simulink model "V2x_TC_IntelligentTrafficSigns_cs"  (Simulink 7.6)
settings: Solver=FixedStepDiscrete  SolverMode=SingleTasking  StartTime=0.0  StopTime=66  FixedStep=1/100  RelTol=1e-3
PreLoadFcn:
  | check_NUTS_defined
  | 
  | assignin('base',['model_closed_' bdroot],false);addpath('../includes_V2x');
InitFcn:
  | PreScanExperimentInitFcn(bdroot)
StopFcn:
  | PreScanExperimentStopFcn(bdroot);
  | 
undefined symbols (not defined in the file or in its folder): acceleration, actorID, actorType, DiscIntNeverNeededParam, GPS_alt, GPS_lat, GPS_long, lightBarInUse, messageID, Newton, publicActorType, Raphson, rotZ, sigbuilder_block, sireneInUse, trafficLightPriority, turnAdvice, velocity, x_ini_VRML, y_ini_VRML, yawRate, z_ini_VRML
libraries: simulink x111, PreScanSimulinkLibrary x7, simulink_extras x4, PreScanUsefulBlocks x3
(part 15 of 16)

== system V2x_TC_IntelligentTrafficSigns_cs/TS_Tcross_2/Infrastructure CAM generator/CAM generation/no ==
  Action Port : ActionPort  PropagateVarSize=During execution  ActionType=else
  Constant : Constant  Value=0
  Out1 : Outport  OutputWhenDisabled=reset  InitialOutput=0
  wires:
  Constant/1 -> Out1/1

== system V2x_TC_IntelligentTrafficSigns_cs/TS_Tcross_2/Infrastructure CAM generator/CAM generation/yes ==
  Action Port : ActionPort  PropagateVarSize=During execution  ActionType=then
  Constant : Constant
  Data Store Write5 : DataStoreWrite  DataStoreName=CAM_time
  Digital Clock1 : DigitalClock  SampleTime=0.01
  Out1 : Outport  OutputWhenDisabled=reset  InitialOutput=0
  wires:
  Digital Clock1/1 -> Data Store Write5/1
  Constant/1 -> Out1/1

== system V2x_TC_IntelligentTrafficSigns_cs/TS_Tcross_3/Infrastructure CAM generator/CAM generation/no ==
  Action Port : ActionPort  PropagateVarSize=During execution  ActionType=else
  Constant : Constant  Value=0
  Out1 : Outport  OutputWhenDisabled=reset  InitialOutput=0
  wires:
  Constant/1 -> Out1/1

== system V2x_TC_IntelligentTrafficSigns_cs/TS_Tcross_3/Infrastructure CAM generator/CAM generation/yes ==
  Action Port : ActionPort  PropagateVarSize=During execution  ActionType=then
  Constant : Constant
  Data Store Write5 : DataStoreWrite  DataStoreName=CAM_time
  Digital Clock1 : DigitalClock  SampleTime=0.01
  Out1 : Outport  OutputWhenDisabled=reset  InitialOutput=0
  wires:
  Digital Clock1/1 -> Data Store Write5/1
  Constant/1 -> Out1/1

== system V2x_TC_IntelligentTrafficSigns_cs/TS_end_restrictions/Infrastructure CAM generator/CAM generation/no ==
  Action Port : ActionPort  PropagateVarSize=During execution  ActionType=else
  Constant : Constant  Value=0
  Out1 : Outport  OutputWhenDisabled=reset  InitialOutput=0
  wires:
  Constant/1 -> Out1/1

== system V2x_TC_IntelligentTrafficSigns_cs/TS_end_restrictions/Infrastructure CAM generator/CAM generation/yes ==
  Action Port : ActionPort  PropagateVarSize=During execution  ActionType=then
  Constant : Constant
  Data Store Write5 : DataStoreWrite  DataStoreName=CAM_time
  Digital Clock1 : DigitalClock  SampleTime=0.01
  Out1 : Outport  OutputWhenDisabled=reset  InitialOutput=0
  wires:
  Digital Clock1/1 -> Data Store Write5/1
  Constant/1 -> Out1/1

== system V2x_TC_IntelligentTrafficSigns_cs/TS_end_restrictions_r/Infrastructure CAM generator/CAM generation/no ==
  Action Port : ActionPort  PropagateVarSize=During execution  ActionType=else
  Constant : Constant  Value=0
  Out1 : Outport  OutputWhenDisabled=reset  InitialOutput=0
  wires:
  Constant/1 -> Out1/1

== system V2x_TC_IntelligentTrafficSigns_cs/TS_end_restrictions_r/Infrastructure CAM generator/CAM generation/yes ==
  Action Port : ActionPort  PropagateVarSize=During execution  ActionType=then
  Constant : Constant
  Data Store Write5 : DataStoreWrite  DataStoreName=CAM_time
  Digital Clock1 : DigitalClock  SampleTime=0.01
  Out1 : Outport  OutputWhenDisabled=reset  InitialOutput=0
  wires:
  Digital Clock1/1 -> Data Store Write5/1
  Constant/1 -> Out1/1

== system V2x_TC_IntelligentTrafficSigns_cs/TS_no_entry/Infrastructure CAM generator/CAM generation/no ==
  Action Port : ActionPort  PropagateVarSize=During execution  ActionType=else
  Constant : Constant  Value=0
  Out1 : Outport  OutputWhenDisabled=reset  InitialOutput=0
  wires:
  Constant/1 -> Out1/1

== system V2x_TC_IntelligentTrafficSigns_cs/TS_no_entry/Infrastructure CAM generator/CAM generation/yes ==
  Action Port : ActionPort  PropagateVarSize=During execution  ActionType=then
  Constant : Constant
  Data Store Write5 : DataStoreWrite  DataStoreName=CAM_time
  Digital Clock1 : DigitalClock  SampleTime=0.01
  Out1 : Outport  OutputWhenDisabled=reset  InitialOutput=0
  wires:
  Digital Clock1/1 -> Data Store Write5/1
  Constant/1 -> Out1/1

== system V2x_TC_IntelligentTrafficSigns_cs/TS_v50_1/Infrastructure CAM generator/CAM generation/no ==
  Action Port : ActionPort  PropagateVarSize=During execution  ActionType=else
  Constant : Constant  Value=0
  Out1 : Outport  OutputWhenDisabled=reset  InitialOutput=0
  wires:
  Constant/1 -> Out1/1

== system V2x_TC_IntelligentTrafficSigns_cs/TS_v50_1/Infrastructure CAM generator/CAM generation/yes ==
  Action Port : ActionPort  PropagateVarSize=During execution  ActionType=then
  Constant : Constant
  Data Store Write5 : DataStoreWrite  DataStoreName=CAM_time
  Digital Clock1 : DigitalClock  SampleTime=0.01
  Out1 : Outport  OutputWhenDisabled=reset  InitialOutput=0
  wires:
  Digital Clock1/1 -> Data Store Write5/1
  Constant/1 -> Out1/1

== system V2x_TC_IntelligentTrafficSigns_cs/TS_v50_2/Infrastructure CAM generator/CAM generation/no ==
  Action Port : ActionPort  PropagateVarSize=During execution  ActionType=else
  Constant : Constant  Value=0
  Out1 : Outport  OutputWhenDisabled=reset  InitialOutput=0
  wires:
  Constant/1 -> Out1/1

== system V2x_TC_IntelligentTrafficSigns_cs/TS_v50_2/Infrastructure CAM generator/CAM generation/yes ==
  Action Port : ActionPort  PropagateVarSize=During execution  ActionType=then
  Constant : Constant
  Data Store Write5 : DataStoreWrite  DataStoreName=CAM_time
  Digital Clock1 : DigitalClock  SampleTime=0.01
  Out1 : Outport  OutputWhenDisabled=reset  InitialOutput=0
  wires:
  Digital Clock1/1 -> Data Store Write5/1
  Constant/1 -> Out1/1

== system V2x_TC_IntelligentTrafficSigns_cs/TS_v50_3/Infrastructure CAM generator/CAM generation/no ==
  Action Port : ActionPort  PropagateVarSize=During execution  ActionType=else
  Constant : Constant  Value=0
  Out1 : Outport  OutputWhenDisabled=reset  InitialOutput=0
  wires:
  Constant/1 -> Out1/1

== system V2x_TC_IntelligentTrafficSigns_cs/TS_v50_3/Infrastructure CAM generator/CAM generation/yes ==
  Action Port : ActionPort  PropagateVarSize=During execution  ActionType=then
  Constant : Constant
  Data Store Write5 : DataStoreWrite  DataStoreName=CAM_time
  Digital Clock1 : DigitalClock  SampleTime=0.01
  Out1 : Outport  OutputWhenDisabled=reset  InitialOutput=0
  wires:
  Digital Clock1/1 -> Data Store Write5/1
  Constant/1 -> Out1/1

== system V2x_TC_IntelligentTrafficSigns_cs/TS_v80_1/Infrastructure CAM generator/CAM generation/no ==
  Action Port : ActionPort  PropagateVarSize=During execution  ActionType=else
  Constant : Constant  Value=0
  Out1 : Outport  OutputWhenDisabled=reset  InitialOutput=0
  wires:
  Constant/1 -> Out1/1

== system V2x_TC_IntelligentTrafficSigns_cs/TS_v80_1/Infrastructure CAM generator/CAM generation/yes ==
  Action Port : ActionPort  PropagateVarSize=During execution  ActionType=then
  Constant : Constant
  Data Store Write5 : DataStoreWrite  DataStoreName=CAM_time
  Digital Clock1 : DigitalClock  SampleTime=0.01
  Out1 : Outport  OutputWhenDisabled=reset  InitialOutput=0
  wires:
  Digital Clock1/1 -> Data Store Write5/1
  Constant/1 -> Out1/1

== system V2x_TC_IntelligentTrafficSigns_cs/TS_v80_3/Infrastructure CAM generator/CAM generation/no ==
  Action Port : ActionPort  PropagateVarSize=During execution  ActionType=else
  Constant : Constant  Value=0
  Out1 : Outport  OutputWhenDisabled=reset  InitialOutput=0
  wires:
  Constant/1 -> Out1/1

== system V2x_TC_IntelligentTrafficSigns_cs/TS_v80_3/Infrastructure CAM generator/CAM generation/yes ==
  Action Port : ActionPort  PropagateVarSize=During execution  ActionType=then
  Constant : Constant
  Data Store Write5 : DataStoreWrite  DataStoreName=CAM_time
  Digital Clock1 : DigitalClock  SampleTime=0.01
  Out1 : Outport  OutputWhenDisabled=reset  InitialOutput=0
  wires:
  Digital Clock1/1 -> Data Store Write5/1
  Constant/1 -> Out1/1

== system V2x_TC_IntelligentTrafficSigns_cs/TS_v80_4/Infrastructure CAM generator/CAM generation/no ==
  Action Port : ActionPort  PropagateVarSize=During execution  ActionType=else
  Constant : Constant  Value=0
  Out1 : Outport  OutputWhenDisabled=reset  InitialOutput=0
  wires:
  Constant/1 -> Out1/1

== system V2x_TC_IntelligentTrafficSigns_cs/TS_v80_4/Infrastructure CAM generator/CAM generation/yes ==
  Action Port : ActionPort  PropagateVarSize=During execution  ActionType=then
  Constant : Constant
  Data Store Write5 : DataStoreWrite  DataStoreName=CAM_time
  Digital Clock1 : DigitalClock  SampleTime=0.01
  Out1 : Outport  OutputWhenDisabled=reset  InitialOutput=0
  wires:
  Digital Clock1/1 -> Data Store Write5/1
  Constant/1 -> Out1/1

== system V2x_TC_IntelligentTrafficSigns_cs/BMW_X5_1/Traffic Controller/Intersection warning controller/Enabled Subsystem/Intersection ==
  hostRotZ : Inport
  hostTurnAdvice : Inport  Port=2
  host GPS lat [deg] : Inport  Port=3
  host GPS long [deg] : Inport  Port=4
  host GPS alt [m] : Inport  Port=5
  GPS_lat : Inport  Port=6
  GPS_long : Inport  Port=7
  GPS_alt : Inport  Port=8
  targetVelocity : Inport  Port=9
  turnAdvice : Inport  Port=10
  rotZ : Inport  Port=11
  actorType : Inport  Port=12
  crossingType : Inport  Port=13
  crossing detection range : Inport  Port=14
  Closest crossing selection : Subsystem
  GPS to XYZ difference (vectorized) : Subsystem
  GPS_alt_1 : InportShadow  Port=8
  GPS_lat_1 : InportShadow  Port=6
  GPS_long_1 : InportShadow  Port=7
  Intersection collision warning controller : Subsystem
  Terminator_2 : Terminator
  cartesian 2 cylindrical : Subsystem
  crossing detection range_1 : InportShadow  Port=14
  hostRotZ_1 : InportShadow  Port=1
  relative heading : Subsystem
  warning left lane : Outport
  warning straight lane : Outport  Port=2
  warning right lane : Outport  Port=3
  wires:
  host GPS lat [deg]/1 -> Closest crossing selection/2(host GPS lat)
  host GPS long [deg]/1 -> Closest crossing selection/3(host GPS long)
  host GPS alt [m]/1 -> Closest crossing selection/4(host GPS alt)
  Closest crossing selection/1(crossing GPS lat) -> GPS to XYZ difference (vectorized)/1(hostLat)
  Closest crossing selection/2(crossing GPS long) -> GPS to XYZ difference (vectorized)/2(hostLong)
  Closest crossing selection/3(crossing GPS alt) -> GPS to XYZ difference (vectorized)/3(hostAlt)
  crossing detection range/1 -> Closest crossing selection/8(crossing detection range)
  crossing detection range_1/1 -> Intersection collision warning controller/3(crossing detection range)
  hostRotZ/1 -> relative heading/2(hostRotZ)
  hostTurnAdvice/1 -> Intersection collision warning controller/2(hostTurnAdvice)
  cartesian 2 cylindrical/1(range) -> Intersection collision warning controller/6(target range)
  relative heading/1(dRotZ) -> Intersection collision warning controller/8(target heading)
  Intersection collision warning controller/1(warning left lane) -> warning left lane/1
  Intersection collision warning controller/2(warning straight lane) -> warning straight lane/1
  Intersection collision warning controller/3(warning right lane) -> warning right lane/1
  GPS to XYZ difference (vectorized)/1(dX) -> cartesian 2 cylindrical/1(dX)
  GPS to XYZ difference (vectorized)/2(dY) -> cartesian 2 cylindrical/2(dY)
  GPS_lat/1 -> Closest crossing selection/5(GPS lat)
  GPS_long/1 -> Closest crossing selection/6(GPS long)
  GPS_alt/1 -> Closest crossing selection/7(GPS alt)
  crossingType/1 -> Closest crossing selection/1(crossingType)
  rotZ/1 -> relative heading/1(rotZ)
  GPS_lat_1/1 -> GPS to XYZ difference (vectorized)/4(lat)
  GPS_long_1/1 -> GPS to XYZ difference (vectorized)/5(long)
  GPS_alt_1/1 -> GPS to XYZ difference (vectorized)/6(alt)
  turnAdvice/1 -> Intersection collision warning controller/4(target intention)
  targetVelocity/1 -> Intersection collision warning controller/5(target velocity)
  actorType/1 -> Intersection collision warning controller/1(actorType)
  GPS to XYZ difference (vectorized)/3(dZ) -> Terminator_2/1
  hostRotZ_1/1 -> cartesian 2 cylindrical/3(hostRotZ)
  cartesian 2 cylindrical/2(angle) -> Intersection collision warning controller/7(target angle)

== system V2x_TC_IntelligentTrafficSigns_cs/BMW_X5_1/Traffic Controller/Intersection warning controller/Enabled Subsystem/sort using ID ==
  IDs : Inport
  in1 : Inport  Port=2
  in2 : Inport  Port=3
  in3 : Inport  Port=4
  in4 : Inport  Port=5
  in5 : Inport  Port=6
  in6 : Inport  Port=7
  in7 : Inport  Port=8
  in8 : Inport  Port=9
  Embedded MATLAB Function : Stateflow chart  TreatAsAtomicUnit=on
  Selector{1..8} (8 alike) : Selector  IndexOptions=Index vector (port)  Indices=[1 3]  OutputSizes=1
  Out1 : Outport
  Out2 : Outport  Port=2
  Out3 : Outport  Port=3
  Out4 : Outport  Port=4
  Out5 : Outport  Port=5
  Out6 : Outport  Port=6
  Out7 : Outport  Port=7
  Out8 : Outport  Port=8
  wires:
  Embedded MATLAB Function/1(y) -> Selector7/2, Selector8/2, Selector6/2, Selector5/2, Selector4/2, Selector3/2, Selector2/2, Selector1/2
  IDs/1 -> Embedded MATLAB Function/1(ids)
  in7/1 -> Selector7/1
  in6/1 -> Selector6/1
  Selector7/1 -> Out7/1
  Selector6/1 -> Out6/1
  in5/1 -> Selector5/1
  in4/1 -> Selector4/1
  in3/1 -> Selector3/1
  in2/1 -> Selector2/1
  Selector5/1 -> Out5/1
  Selector4/1 -> Out4/1
  Selector3/1 -> Out3/1
  Selector2/1 -> Out2/1
  Selector1/1 -> Out1/1
  in1/1 -> Selector1/1
  in8/1 -> Selector8/1
  Selector8/1 -> Out8/1

== system V2x_TC_IntelligentTrafficSigns_cs/BMW_X5_1/Traffic Controller/Road accident detection controller/Compute distance to road accident/If Action Subsystem ==
  conditions : Inport
  GPS lat1 : Inport  Port=2
  GPS long1 : Inport  Port=3
  GPS alt1 : Inport  Port=4
  host_GPS_lat : Inport  Port=5
  host_GPS_long : Inport  Port=6
  host_GPS_alt : Inport  Port=7
  Action Port : ActionPort  PropagateVarSize=During execution  ActionType=then
  GPS to distance : Subsystem
  Product7 : Product  RndMeth=Zero
  Product8 : Product  RndMeth=Zero
  Product9 : Product  RndMeth=Zero
  Sum6 : Sum  Inputs=+
  Sum7 : Sum  Inputs=+
  Sum8 : Sum  Inputs=+
  distance : Outport  InitialOutput=0
  wires:
  conditions/1 -> Product7/1, Product9/1, Product8/1
  GPS lat1/1 -> Product7/2
  GPS long1/1 -> Product8/2
  GPS alt1/1 -> Product9/2
  Product7/1 -> Sum6/1
  Product8/1 -> Sum7/1
  Product9/1 -> Sum8/1
  Sum6/1 -> GPS to distance/4(lat)
  Sum7/1 -> GPS to distance/5(long)
  Sum8/1 -> GPS to distance/6(alt)
  host_GPS_lat/1 -> GPS to distance/1(hostLat)
  host_GPS_long/1 -> GPS to distance/2(hostLong)
  host_GPS_alt/1 -> GPS to distance/3(hostAlt)
  GPS to distance/1(dist) -> distance/1

== system V2x_TC_IntelligentTrafficSigns_cs/BMW_X5_1/Traffic Controller/Road accident detection controller/Compute distance to road accident/If Action Subsystem1 ==
  Action Port : ActionPort  PropagateVarSize=During execution  ActionType=else
  Constant : Constant  Value=0
  distance : Outport  InitialOutput=0
  wires:
  Constant/1 -> distance/1

== system V2x_TC_IntelligentTrafficSigns_cs/BMW_X5_1/Traffic Controller/Traffic signs and  traffic lights controller/Enabled Subsystem/GPS to XYZ difference (vectorized) ==
  hostLat : Inport
  hostLong : Inport  Port=2
  hostAlt : Inport  Port=3
  lat : Inport  Port=4
  long : Inport  Port=5
  alt : Inport  Port=6
  Add : Sum  Inputs=+-
  Add1 : Sum  Inputs=+-
  Add2 : Sum  Inputs=+-
  Add3 : Sum  Inputs=+++
  Product{,1..4} (5 alike) : Product  RndMeth=Zero
  d2r : Gain  Gain=pi/180
  d2r1 : Gain  Gain=pi/180
  mean Earth radius (latitudal) : Constant  Value=6335439.8775
  mean Earth radius (longitudal) : Constant  Value=6378137.55
  dist_sq : Outport
  dX : Outport  Port=2
  dY : Outport  Port=3
  dZ : Outport  Port=4
  wires:
  Add3/1 -> dist_sq/1
  Product4/1 -> Add3/3
  Product3/1 -> Add3/2
  Product/1 -> Add3/1
  Add2/1 -> Product4/1, Product4/2, dZ/1
  Product1/1 -> Product3/2, Product3/1, dX/1
  Product2/1 -> Product/2, Product/1, dY/1
  mean Earth radius (latitudal)/1 -> Product2/2
  mean Earth radius (longitudal)/1 -> Product1/2
  d2r1/1 -> Product1/1
  d2r/1 -> Product2/1
  Add1/1 -> d2r1/1
  Add/1 -> d2r/1
  hostAlt/1 -> Add2/2
  alt/1 -> Add2/1
  hostLong/1 -> Add1/2
  long/1 -> Add1/1
  hostLat/1 -> Add/2
  lat/1 -> Add/1

== system V2x_TC_IntelligentTrafficSigns_cs/BMW_X5_1/Traffic Controller/Traffic signs and  traffic lights controller/Enabled Subsystem/distance condition ==
  distance_squared : Inport
  hostVelocity : Inport  Port=2
  If : If  IfExpression=u1 ~= 0
  If Action Subsystem : Subsystem  TreatAsAtomicUnit=on
  If Action Subsystem1 : Subsystem  TreatAsAtomicUnit=on
  Merge : Merge
  Product3 : Product  RndMeth=Zero
  Product4 : Product  RndMeth=Zero
  Relational Operator1 : RelationalOperator  Operator=<  OutDataTypeStr=boolean
  headway time : Constant  Value=3
  dist_cond : Outport
  wires:
  Product3/1 -> If Action Subsystem1/1(headway time), Relational Operator1/2
  distance_squared/1 -> If Action Subsystem1/2(d2), If Action Subsystem/1(d2)
  Relational Operator1/1 -> dist_cond/1
  Merge/1 -> Relational Operator1/1
  If Action Subsystem1/1(t2) -> Merge/2
  If Action Subsystem/1(t2) -> Merge/1
  Product4/1 -> If Action Subsystem/2(v2)
  If/2 -> If Action Subsystem1/Action
  If/1 -> If Action Subsystem/Action
  hostVelocity/1 -> Product4/2, Product4/1, If/1
  headway time/1 -> Product3/1, Product3/2

== system V2x_TC_IntelligentTrafficSigns_cs/BMW_X5_1/Traffic Controller/Traffic signs and  traffic lights controller/Enabled Subsystem/heading condition ==
  hostRotZ : Inport
  rotZ : Inport  Port=2
  Abs : Abs
  Add : Sum  Inputs=+-
  Add1 : Sum  Inputs=-+
  Bias : Bias  Bias=180
  Constant : Constant  Value=360
  Constant1 : Constant  Value=360
  Logical Operator : Logic  Operator=OR  AllPortsSameDT=off  OutDataTypeStr=boolean
  Math Function : Math  Operator=mod  SaturateOnIntegerOverflow=on
  Relational Operator : RelationalOperator  Operator=<  OutDataTypeStr=boolean
  Relational Operator1 : RelationalOperator  Operator=>  OutDataTypeStr=boolean
  heading difference : Constant  Value=45
  head_cond : Outport
  wires:
  Logical Operator/1 -> head_cond/1
  Relational Operator1/1 -> Logical Operator/2
  Relational Operator/1 -> Logical Operator/1
  Constant1/1 -> Add1/2
  Add1/1 -> Relational Operator1/2
  heading difference/1 -> Add1/1, Relational Operator/2
  Abs/1 -> Relational Operator1/1, Relational Operator/1
  Math Function/1 -> Abs/1
  hostRotZ/1 -> Add/2
  rotZ/1 -> Add/1
  Bias/1 -> Math Function/1
  Constant/1 -> Math Function/2
  Add/1 -> Bias/1

== system V2x_TC_IntelligentTrafficSigns_cs/BMW_X5_1/Traffic Controller/Traffic signs and  traffic lights controller/Enabled Subsystem/major object selection lights ==
  conditions : Inport
  messagesID : Inport  Port=2
  traffic lights priorities : Inport  Port=3
  If : If  IfExpression=u1
  If Action Subsystem : Subsystem  TreatAsAtomicUnit=on
  If Action Subsystem1 : Subsystem  TreatAsAtomicUnit=on
  Logical Operator1 : Logic  Operator=OR  Inputs=1  AllPortsSameDT=off  OutDataTypeStr=boolean
  Merge : Merge
  Merge2 : Merge
  Traffic Lights Warning : Subsystem
  Warning : Outport
  MessageID : Outport  Port=2
  Priority : Outport  Port=3
  wires:
  Traffic Lights Warning/1(Warning) -> Warning/1
  Merge/1 -> Traffic Lights Warning/1(trafficLightPriority), Priority/1
  Merge2/1 -> MessageID/1
  If Action Subsystem/2(Priority) -> Merge/1
  If Action Subsystem/1(Message) -> Merge2/1
  If Action Subsystem1/1(Message) -> Merge2/2
  If Action Subsystem1/2(Priority) -> Merge/2
  traffic lights priorities/1 -> If Action Subsystem/3(traffic lights priorities)
  messagesID/1 -> If Action Subsystem/2(messagesID)
  conditions/1 -> If Action Subsystem/1(cond), Logical Operator1/1
  If/2 -> If Action Subsystem1/Action
  If/1 -> If Action Subsystem/Action
  Logical Operator1/1 -> If/1

== system V2x_TC_IntelligentTrafficSigns_cs/BMW_X5_1/Traffic Controller/Traffic signs and  traffic lights controller/Enabled Subsystem/major object selection signs ==
  conditions : Inport
  hostVelocity : Inport  Port=2
  messagesID : Inport  Port=3
  If : If  IfExpression=u1
  If Action Subsystem : Subsystem  TreatAsAtomicUnit=on
  If Action Subsystem1 : Subsystem  TreatAsAtomicUnit=on
  Logical Operator1 : Logic  Operator=OR  Inputs=1  AllPortsSameDT=off  OutDataTypeStr=boolean
  Merge : Merge
  Merge1 : Merge
  Warning : Outport
  MessageID : Outport  Port=2
  wires:
  hostVelocity/1 -> If Action Subsystem/3(hostVelocity)
  Merge/1 -> MessageID/1
  Merge1/1 -> Warning/1
  If Action Subsystem1/1(Warning) -> Merge1/2
  If Action Subsystem/1(Warning) -> Merge1/1
  If Action Subsystem/2(Message) -> Merge/1
  If Action Subsystem1/2(Message) -> Merge/2
  messagesID/1 -> If Action Subsystem/2(messagesID)
  conditions/1 -> If Action Subsystem/1(cond), Logical Operator1/1
  If/2 -> If Action Subsystem1/Action
  If/1 -> If Action Subsystem/Action
  Logical Operator1/1 -> If/1

== system V2x_TC_IntelligentTrafficSigns_cs/BMW_X5_1/Traffic Controller/Traffic signs and  traffic lights controller/Enabled Subsystem/orientation condition ==
  hostRotZ : Inport
  dX : Inport  Port=2
  dY : Inport  Port=3
  dZ : Inport  Port=4
  Abs : Abs
  Gain : Gain  Gain=pi/180
  Relational Operator : RelationalOperator  Operator=<  OutDataTypeStr=boolean
  Terminator : Terminator
  Trigonometric Function : Trigonometry  Operator=atan2
  d2r : Gain  Gain=pi/180
  orientation limit : Constant  Value=45
  to local reference system : Subsystem
  orient_cond : Outport
  wires:
  to local reference system/2(dx) -> Trigonometric Function/2
  to local reference system/1(dy) -> Trigonometric Function/1
  dY/1 -> to local reference system/3(dY)
  dX/1 -> to local reference system/2(dX)
  Gain/1 -> to local reference system/1(rotZ)
  hostRotZ/1 -> Gain/1
  Trigonometric Function/1 -> Abs/1
  Relational Operator/1 -> orient_cond/1
  d2r/1 -> Relational Operator/2
  Abs/1 -> Relational Operator/1
  orientation limit/1 -> d2r/1
  dZ/1 -> Terminator/1

== system V2x_TC_IntelligentTrafficSigns_cs/BMW_X5_1/Traffic Controller/Traffic signs and  traffic lights controller/Enabled Subsystem/right road side condition ==
  hostRotZ : Inport
  dX : Inport  Port=2
  dY : Inport  Port=3
  Compare To Zero : simulink/Logic and Bit Operations/Compare To Zero  SystemSampleTime=-1  relop=<  LogicOutDataTypeMode=boolean
  Gain : Gain  Gain=pi/180
  to local reference system : Subsystem
  orient_cond : Outport
  wires:
  Compare To Zero/1 -> orient_cond/1
  hostRotZ/1 -> Gain/1
  Gain/1 -> to local reference system/1(rotZ)
  dX/1 -> to local reference system/2(dX)
  dY/1 -> to local reference system/3(dY)
  to local reference system/1(dy) -> Compare To Zero/1

== system V2x_TC_IntelligentTrafficSigns_cs/BMW_X5_1/Trajectories/ElapsedTrack1/MuxState/Substitute 90 & 270 deg (and its plurals) ==
  Angle [rad] : Inport
  270 deg detection : simulink/Model Verification/Check  Static Gap  Description=u <= 269.5 || 270.5 <= u  SystemSampleTime=-1  max=270.5  max_included=on  min=269.5  min_included=on  enabled=on  stopWhenAssertionFail=off  export=on  icon=graphic
  90 deg detection : simulink/Model Verification/Check  Static Gap  Description=u <= 89.5 || 90.5 <= u  SystemSampleTime=-1  max=90.5  max_included=on  min=89.5  min_included=on  enabled=on  stopWhenAssertionFail=off  export=on  icon=graphic
  Angle 0-360 deg limiter : Subsystem
  Degrees to Radians : simulink_extras/Transformations/Degrees to Radians  SystemSampleTime=-1
  Radians to Degrees : simulink_extras/Transformations/Radians to Degrees  SystemSampleTime=-1
  Substitution  Value : Constant  Value=269.5
  Substitution Value : Constant  Value=89.5
  Switch : Switch  Threshold=0.5
  Switch1 : Switch  Threshold=0.5
  Subst Angle [rad] : Outport
  wires:
  Angle [rad]/1 -> Radians to Degrees/1
  Degrees to Radians/1 -> Subst Angle [rad]/1
  Radians to Degrees/1 -> Angle 0-360 deg limiter/1(Angle [deg])
  Switch1/1 -> Degrees to Radians/1
  Substitution  Value/1 -> Switch1/3
  270 deg detection/1 -> Switch1/2
  Switch/1 -> Switch1/1, 270 deg detection/1
  Substitution Value/1 -> Switch/3
  90 deg detection/1 -> Switch/2
  Angle 0-360 deg limiter/1(LimAngle [deg]) -> 90 deg detection/1, Switch/1

== system V2x_TC_IntelligentTrafficSigns_cs/BMW_X5_1/Trajectories/Traj2State1/Traj2State2/MuxState ==
  x [m] : Inport
  y [m] : Inport  Port=2
  z [m] : Inport  Port=3
  dx [m/s] : Inport  Port=4
  dy [m/s] : Inport  Port=5
  dz [m/s] : Inport  Port=6
  R [rad] : Inport  Port=7
  P [rad] : Inport  Port=8
  Y [rad] : Inport  Port=9
  Mux : Mux  Inputs=9
  state : Outport
  wires:
  z [m]/1 -> Mux/3
  y [m]/1 -> Mux/2
  x [m]/1 -> Mux/1
  Y [rad]/1 -> Mux/9
  P [rad]/1 -> Mux/8
  R [rad]/1 -> Mux/7
  dz [m/s]/1 -> Mux/6
  dy [m/s]/1 -> Mux/5
  dx [m/s]/1 -> Mux/4
  Mux/1 -> state/1

== system V2x_TC_IntelligentTrafficSigns_cs/BMW_X5_1/Trajectories/Traj2State1/Traj2State2/Traj2MuxState_Ext ==
  Traj : Inport
  Terminator{1..9} (9 alike) : Terminator
  demux : Subsystem
  x [m] : Outport
  y [m] : Outport  Port=2
  z [m] : Outport  Port=3
  dx [m/s] : Outport  Port=4
  dy [m/s] : Outport  Port=5
  dz [m/s] : Outport  Port=6
  R [rad] : Outport  Port=7
  P [rad] : Outport  Port=8
  Y [rad] : Outport  Port=9
  wires:
  demux/9(Y) -> Y [rad]/1
  demux/7(R) -> R [rad]/1
  demux/5(vely) -> dy [m/s]/1
  demux/4(velx) -> dx [m/s]/1
  demux/10(velR) -> Terminator4/1
  demux/11(velP) -> Terminator5/1
  demux/12(velY) -> Terminator1/1
  demux/13(accx) -> Terminator8/1
  demux/14(accy) -> Terminator9/1
  demux/15(accz) -> Terminator7/1
  demux/18(accY) -> Terminator2/1
  demux/16(accR) -> Terminator3/1
  demux/17(accP) -> Terminator6/1
  demux/3(z) -> z [m]/1
  Traj/1 -> demux/1(traj)
  demux/6(velz) -> dz [m/s]/1
  demux/1(x) -> x [m]/1
  demux/2(y) -> y [m]/1
  demux/8(P) -> P [rad]/1

== system V2x_TC_IntelligentTrafficSigns_cs/BMW_X5_1/vehicle CAM generator/CAM generation/maybe/If Action Subsystem ==
  x [m] : Inport
  y [m] : Inport  Port=2
  z [m] : Inport  Port=3
  rotZ [deg] : Inport  Port=4
  velocity [m/s] : Inport  Port=5
  Action Port : ActionPort  PropagateVarSize=During execution  ActionType=then
  Constant : Constant
  Data Store Write : DataStoreWrite  DataStoreName=CAM_velocity
  Data Store Write1 : DataStoreWrite  DataStoreName=CAM_heading
  Data Store Write2 : DataStoreWrite  DataStoreName=CAM_x
  Data Store Write3 : DataStoreWrite  DataStoreName=CAM_y
  Data Store Write4 : DataStoreWrite  DataStoreName=CAM_z
  Data Store Write5 : DataStoreWrite  DataStoreName=CAM_time
  Digital Clock1 : DigitalClock  SampleTime=0.01
  Out1 : Outport  InitialOutput=0
  wires:
  Constant/1 -> Out1/1
  velocity [m/s]/1 -> Data Store Write/1
  rotZ [deg]/1 -> Data Store Write1/1
  x [m]/1 -> Data Store Write2/1
  y [m]/1 -> Data Store Write3/1
  z [m]/1 -> Data Store Write4/1
  Digital Clock1/1 -> Data Store Write5/1

== system V2x_TC_IntelligentTrafficSigns_cs/BMW_X5_1/vehicle CAM generator/CAM generation/maybe/If Action Subsystem1 ==
  Action Port : ActionPort  PropagateVarSize=During execution  ActionType=else
  Constant : Constant  Value=0
  Out1 : Outport  InitialOutput=0
  wires:
  Constant/1 -> Out1/1

== system V2x_TC_IntelligentTrafficSigns_cs/BMW_X5_1/Traffic Controller/Intersection warning controller/Enabled Subsystem/Intersection/Closest crossing selection ==
  crossingType : Inport
  host GPS lat : Inport  Port=2
  host GPS long : Inport  Port=3
  host GPS alt : Inport  Port=4
  GPS lat : Inport  Port=5
  GPS long : Inport  Port=6
  GPS alt : Inport  Port=7
  crossing detection range : Inport  Port=8
  GPS alt1 : InportShadow  Port=7
  GPS lat1 : InportShadow  Port=5
  GPS long1 : InportShadow  Port=6
  GPS to XYZ difference (vectorized) : Subsystem
  If : If  NumInputs=2  IfExpression=u1 ~= u2
  If Action Subsystem : Subsystem  TreatAsAtomicUnit=on
  If Action Subsystem1 : Subsystem  TreatAsAtomicUnit=on
  Logical Operator : Logic  AllPortsSameDT=off  OutDataTypeStr=boolean
  Merge : Merge
  Merge1 : Merge
  Merge2 : Merge
  MinMax : MinMax
  Product : Product  RndMeth=Zero
  Relational Operator : RelationalOperator  Operator=<  OutDataTypeStr=boolean
  Switch : Switch  Criteria=u2 > Threshold
  crossing GPS lat : Outport
  crossing GPS long : Outport  Port=2
  crossing GPS alt : Outport  Port=3
  wires:
  GPS to XYZ difference (vectorized)/1(dist_sq) -> Relational Operator/1, Switch/1, If Action Subsystem/2(dist_sq)
  crossing detection range/1 -> Product/2, Product/1
  Product/1 -> Relational Operator/2, Switch/3, If/2
  Relational Operator/1 -> Logical Operator/1
  crossingType/1 -> Logical Operator/2
  Logical Operator/1 -> Switch/2
  Switch/1 -> MinMax/1
  MinMax/1 -> If Action Subsystem/1(min), If/1
  host GPS lat/1 -> GPS to XYZ difference (vectorized)/1(hostLat)
  host GPS long/1 -> GPS to XYZ difference (vectorized)/2(hostLong)
  host GPS alt/1 -> GPS to XYZ difference (vectorized)/3(hostAlt)
  GPS lat/1 -> GPS to XYZ difference (vectorized)/4(lat)
  GPS long/1 -> GPS to XYZ difference (vectorized)/5(long)
  GPS alt/1 -> GPS to XYZ difference (vectorized)/6(alt)
  If/1 -> If Action Subsystem/Action
  If/2 -> If Action Subsystem1/Action
  GPS lat1/1 -> If Action Subsystem/3(GPS lat1)
  GPS long1/1 -> If Action Subsystem/4(GPS long1)
  GPS alt1/1 -> If Action Subsystem/5(GPS alt1)
  If Action Subsystem/1(GPS lat) -> Merge/1
  If Action Subsystem/2(GPS long) -> Merge2/1
  If Action Subsystem/3(GPS alt) -> Merge1/1
  If Action Subsystem1/1(GPS lat) -> Merge/2
  If Action Subsystem1/2(GPS long) -> Merge2/2
  If Action Subsystem1/3(GPS alt) -> Merge1/2
  Merge2/1 -> crossing GPS long/1
  Merge/1 -> crossing GPS lat/1
  Merge1/1 -> crossing GPS alt/1

== system V2x_TC_IntelligentTrafficSigns_cs/BMW_X5_1/Traffic Controller/Intersection warning controller/Enabled Subsystem/Intersection/GPS to XYZ difference (vectorized) ==
  hostLat : Inport
  hostLong : Inport  Port=2
  hostAlt : Inport  Port=3
  lat : Inport  Port=4
  long : Inport  Port=5
  alt : Inport  Port=6
  Add : Sum  Inputs=+-
  Add1 : Sum  Inputs=+-
  Add2 : Sum  Inputs=+-
  Product1 : Product  RndMeth=Zero
  Product2 : Product  RndMeth=Zero
  d2r : Gain  Gain=pi/180
  d2r1 : Gain  Gain=pi/180
  mean Earth radius (latitudal) : Constant  Value=6335439.8775
  mean Earth radius (longitudal) : Constant  Value=6378137.55
  dX : Outport
  dY : Outport  Port=2
  dZ : Outport  Port=3
  wires:
  lat/1 -> Add/1
  hostLat/1 -> Add/2
  long/1 -> Add1/1
  hostLong/1 -> Add1/2
  alt/1 -> Add2/1
  hostAlt/1 -> Add2/2
  Add/1 -> d2r/1
  Add1/1 -> d2r1/1
  d2r/1 -> Product2/1
  d2r1/1 -> Product1/1
  mean Earth radius (longitudal)/1 -> Product1/2
  mean Earth radius (latitudal)/1 -> Product2/2
  Product2/1 -> dY/1
  Product1/1 -> dX/1
  Add2/1 -> dZ/1

== system V2x_TC_IntelligentTrafficSigns_cs/BMW_X5_1/Traffic Controller/Intersection warning controller/Enabled Subsystem/Intersection/Intersection collision warning controller ==
  actorType : Inport
  hostTurnAdvice : Inport  Port=2
  crossing detection range : Inport  Port=3
  target intention : Inport  Port=4
  target velocity : Inport  Port=5
  target range : Inport  Port=6
  target angle : Inport  Port=7
  target heading : Inport  Port=8
  For Each Subsystem : Subsystem  TreatAsAtomicUnit=on
  Logical Operator : Logic  Operator=OR  Inputs=1  AllPortsSameDT=off  OutDataTypeStr=boolean
  Logical Operator1 : Logic  Operator=OR  Inputs=1  AllPortsSameDT=off  OutDataTypeStr=boolean
  Logical Operator2 : Logic  Operator=OR  Inputs=1  AllPortsSameDT=off  OutDataTypeStr=boolean
  host intention translation : Subsystem
  warning left lane : Outport
  warning straight lane : Outport  Port=2
  warning right lane : Outport  Port=3
  wires:
  hostTurnAdvice/1 -> host intention translation/1(host intention)
  For Each Subsystem/1(warning left lane) -> Logical Operator/1
  For Each Subsystem/2(warning straight lane) -> Logical Operator1/1
  For Each Subsystem/3(warning right lane) -> Logical Operator2/1
  actorType/1 -> For Each Subsystem/1(actorType)
  host intention translation/1(WM) -> For Each Subsystem/2(WM)
  crossing detection range/1 -> For Each Subsystem/3(Rcross)
  target range/1 -> For Each Subsystem/4(target range)
  target angle/1 -> For Each Subsystem/5(target angle)
  target intention/1 -> For Each Subsystem/6(target intention)
  target heading/1 -> For Each Subsystem/7(target heading)
  Logical Operator/1 -> warning left lane/1
  Logical Operator1/1 -> warning straight lane/1
  Logical Operator2/1 -> warning right lane/1
  target velocity/1 -> For Each Subsystem/8(targetVelocity)

== system V2x_TC_IntelligentTrafficSigns_cs/BMW_X5_1/Traffic Controller/Intersection warning controller/Enabled Subsystem/Intersection/cartesian 2 cylindrical ==
  dX : Inport
  dY : Inport  Port=2
  hostRotZ : Inport  Port=3
  Add : Sum  Inputs=+-
  Complex to Magnitude-Angle1 : ComplexToMagnitudeAngle
  Real-Imag to Complex1 : RealImagToComplex
  r2d : Gain  Gain=180/pi
  range : Outport
  angle : Outport  Port=2
  wires:
  Real-Imag to Complex1/1 -> Complex to Magnitude-Angle1/1
  dX/1 -> Real-Imag to Complex1/1
  Complex to Magnitude-Angle1/1 -> range/1  "range"
  dY/1 -> Real-Imag to Complex1/2
  Complex to Magnitude-Angle1/2 -> r2d/1  "angle"
  r2d/1 -> Add/1
  hostRotZ/1 -> Add/2
  Add/1 -> angle/1

== system V2x_TC_IntelligentTrafficSigns_cs/BMW_X5_1/Traffic Controller/Intersection warning controller/Enabled Subsystem/Intersection/relative heading ==
  rotZ : Inport
  hostRotZ : Inport  Port=2
  Add : Sum  Inputs=+-
  Constant : Constant  Value=360
  Math Function : Math  Operator=mod  SaturateOnIntegerOverflow=on
  dRotZ : Outport
  wires:
  Constant/1 -> Math Function/2
  rotZ/1 -> Add/1
  hostRotZ/1 -> Add/2
  Add/1 -> Math Function/1
  Math Function/1 -> dRotZ/1

== system V2x_TC_IntelligentTrafficSigns_cs/BMW_X5_1/Traffic Controller/Road accident detection controller/Compute distance to road accident/If Action Subsystem/GPS to distance ==
  hostLat : Inport
  hostLong : Inport  Port=2
  hostAlt : Inport  Port=3
  lat : Inport  Port=4
  long : Inport  Port=5
  alt : Inport  Port=6
  Add : Sum  Inputs=+-
  Add1 : Sum  Inputs=+-
  Add2 : Sum  Inputs=+-
  Add3 : Sum  Inputs=+++
  Product{,1..4} (5 alike) : Product  RndMeth=Zero
  Sqrt : Sqrt  AlgorithmType=Newton-Raphson  SaturateOnIntegerOverflow=on
  d2r : Gain  Gain=pi/180
  d2r1 : Gain  Gain=pi/180
  mean Earth radius (latitudal) : Constant  Value=6335439.8775
  mean Earth radius (longitudal) : Constant  Value=6378137.55
  dist : Outport
  wires:
  lat/1 -> Add/1
  hostLat/1 -> Add/2
  long/1 -> Add1/1
  hostLong/1 -> Add1/2
  alt/1 -> Add2/1
  hostAlt/1 -> Add2/2
  Add/1 -> d2r/1
  Add1/1 -> d2r1/1
  d2r/1 -> Product2/1
  d2r1/1 -> Product1/1
  mean Earth radius (longitudal)/1 -> Product1/2
  mean Earth radius (latitudal)/1 -> Product2/2
  Product2/1 -> Product/1, Product/2
  Product1/1 -> Product3/1, Product3/2
  Add2/1 -> Product4/2, Product4/1
  Product/1 -> Add3/1
  Product3/1 -> Add3/2
  Product4/1 -> Add3/3
  Add3/1 -> Sqrt/1
  Sqrt/1 -> dist/1

== system V2x_TC_IntelligentTrafficSigns_cs/BMW_X5_1/Traffic Controller/Traffic signs and  traffic lights controller/Enabled Subsystem/distance condition/If Action Subsystem ==
  d2 : Inport
  v2 : Inport  Port=2
  Action Port : ActionPort  PropagateVarSize=During execution  ActionType=then
  Divide : Product  Inputs=*/
  t2 : Outport
  wires:
  Divide/1 -> t2/1
  v2/1 -> Divide/2
  d2/1 -> Divide/1

== system V2x_TC_IntelligentTrafficSigns_cs/BMW_X5_1/Traffic Controller/Traffic signs and  traffic lights controller/Enabled Subsystem/distance condition/If Action Subsystem1 ==
  headway time : Inport
  d2 : Inport  Port=2
  Action Port : ActionPort  PropagateVarSize=During execution  ActionType=else
  Add : Sum
  Gain : Gain  Gain=0
  t2 : Outport
  wires:
  Add/1 -> t2/1
  headway time/1 -> Add/2
  Gain/1 -> Add/1
  d2/1 -> Gain/1

== system V2x_TC_IntelligentTrafficSigns_cs/BMW_X5_1/Traffic Controller/Traffic signs and  traffic lights controller/Enabled Subsystem/major object selection lights/If Action Subsystem ==
  cond : Inport
  messagesID : Inport  Port=2
  traffic lights priorities : Inport  Port=3
  Action Port : ActionPort  PropagateVarSize=During execution  ActionType=then
  Reshape1 : Reshape  OutputDimensionality=Column vector (2-D)
  Reshape2 : Reshape  OutputDimensionality=Column vector (2-D)
  Reshape3 : Reshape  OutputDimensionality=Column vector (2-D)
  Selector : Selector  NumberOfDimensions=2  InputPortWidth=3  IndexOptions=Index vector (port),Index vector (dialog)  Indices=[1 3],1  OutputSizes=1,1
  Selector1 : Selector  NumberOfDimensions=2  InputPortWidth=3  IndexOptions=Index vector (port),Index vector (dialog)  Indices=[1 3],1  OutputSizes=1,1
  While Iterator Subsystem : Subsystem  TreatAsAtomicUnit=on
  Message : Outport  InitialOutput=0
  Priority : Outport  Port=2  InitialOutput=0
  wires:
  Selector1/1 -> Priority/1
  Reshape3/1 -> Selector1/1
  traffic lights priorities/1 -> Reshape3/1
  messagesID/1 -> Reshape2/1
  While Iterator Subsystem/1(idx) -> Selector1/2, Selector/2
  Reshape2/1 -> Selector/1
  Reshape1/1 -> While Iterator Subsystem/1(cond)
  Selector/1 -> Message/1
  cond/1 -> Reshape1/1

== system V2x_TC_IntelligentTrafficSigns_cs/BMW_X5_1/Traffic Controller/Traffic signs and  traffic lights controller/Enabled Subsystem/major object selection lights/If Action Subsystem1 ==
  Action Port : ActionPort  PropagateVarSize=During execution  ActionType=else
  Constant1 : Constant  Value=0
  Constant2 : Constant  Value=0
  Message : Outport  InitialOutput=0
  Priority : Outport  Port=2  InitialOutput=0
  wires:
  Constant2/1 -> Priority/1
  Constant1/1 -> Message/1

== system V2x_TC_IntelligentTrafficSigns_cs/BMW_X5_1/Traffic Controller/Traffic signs and  traffic lights controller/Enabled Subsystem/major object selection lights/Traffic Lights Warning ==
  trafficLightPriority : Inport
  Data Type Conversion : DataTypeConversion
  Logical Operator : Logic  Operator=OR  AllPortsSameDT=off  OutDataTypeStr=boolean
  if Orange light : simulink/Logic and Bit Operations/Compare To Constant  SystemSampleTime=-1  relop===  const=2  LogicOutDataTypeMode=uint8  ZeroCross=off
  if Red light : simulink/Logic and Bit Operations/Compare To Constant  SystemSampleTime=-1  relop===  const=1  LogicOutDataTypeMode=uint8  ZeroCross=off
  Warning : Outport
  wires:
  trafficLightPriority/1 -> if Orange light/1, if Red light/1
  Data Type Conversion/1 -> Warning/1
  Logical Operator/1 -> Data Type Conversion/1
  if Red light/1 -> Logical Operator/2
  if Orange light/1 -> Logical Operator/1

== system V2x_TC_IntelligentTrafficSigns_cs/BMW_X5_1/Traffic Controller/Traffic signs and  traffic lights controller/Enabled Subsystem/major object selection signs/If Action Subsystem ==
  cond : Inport
  messagesID : Inport  Port=2
  hostVelocity : Inport  Port=3
  Action Port : ActionPort  PropagateVarSize=During execution  ActionType=then
  Reshape1 : Reshape  OutputDimensionality=Column vector (2-D)
  Reshape2 : Reshape  OutputDimensionality=Column vector (2-D)
  Selector : Selector  NumberOfDimensions=2  InputPortWidth=3  IndexOptions=Index vector (port),Index vector (dialog)  Indices=[1 3],1  OutputSizes=1,1
  Selector1 : Selector  NumberOfDimensions=2  InputPortWidth=3  IndexOptions=Index vector (port),Index vector (dialog)  Indices=[1 3],1  OutputSizes=1,1
  Traffic signs warning : Subsystem
  While Iterator Subsystem : Subsystem  TreatAsAtomicUnit=on
  Warning : Outport
  Message : Outport  Port=2  InitialOutput=0
  wires:
  Selector1/1 -> Traffic signs warning/1(MessageID)
  Traffic signs warning/1(Warning) -> Warning/1
  hostVelocity/1 -> Traffic signs warning/2(hostVelocity)
  messagesID/1 -> Reshape2/1
  While Iterator Subsystem/1(idx) -> Selector1/2, Selector/2
  Reshape2/1 -> Selector1/1, Selector/1
  Reshape1/1 -> While Iterator Subsystem/1(cond)
  Selector/1 -> Message/1
  cond/1 -> Reshape1/1

== system V2x_TC_IntelligentTrafficSigns_cs/BMW_X5_1/Traffic Controller/Traffic signs and  traffic lights controller/Enabled Subsystem/major object selection signs/If Action Subsystem1 ==
  Action Port : ActionPort  PropagateVarSize=During execution  ActionType=else
  Constant1 : Constant  Value=0
  Constant2 : Constant  Value=0
  Warning : Outport  InitialOutput=0
  Message : Outport  Port=2  InitialOutput=0
  wires:
  Constant2/1 -> Warning/1
  Constant1/1 -> Message/1

== system V2x_TC_IntelligentTrafficSigns_cs/BMW_X5_1/Traffic Controller/Traffic signs and  traffic lights controller/Enabled Subsystem/orientation condition/to local reference system ==
  rotZ : Inport
  dX : Inport  Port=2
  dY : Inport  Port=3
  Add : Sum
  Add1 : Sum  Inputs=+-
  Product{,1..3} (4 alike) : Product  RndMeth=Zero
  Trigonometric Function : Trigonometry
  Trigonometric Function1 : Trigonometry  Operator=cos
  dy : Outport
  dx : Outport  Port=2
  wires:
  Add1/1 -> dy/1
  Add/1 -> dx/1
  Product3/1 -> Add1/2
  Product2/1 -> Add1/1
  Product/1 -> Add/2
  Product1/1 -> Add/1
  dY/1 -> Product2/2, Product1/2
  Trigonometric Function/1 -> Product3/1, Product1/1
  dX/1 -> Product3/2, Product/2
  Trigonometric Function1/1 -> Product2/1, Product/1
  rotZ/1 -> Trigonometric Function1/1, Trigonometric Function/1

== system V2x_TC_IntelligentTrafficSigns_cs/BMW_X5_1/Traffic Controller/Traffic signs and  traffic lights controller/Enabled Subsystem/right road side condition/to local reference system ==
  rotZ : Inport
  dX : Inport  Port=2
  dY : Inport  Port=3
  Add1 : Sum  Inputs=+-
  Product2 : Product  RndMeth=Zero
  Product3 : Product  RndMeth=Zero
  Trigonometric Function : Trigonometry
  Trigonometric Function1 : Trigonometry  Operator=cos
  dy : Outport
  wires:
  rotZ/1 -> Trigonometric Function/1, Trigonometric Function1/1
  Trigonometric Function1/1 -> Product2/1
  dX/1 -> Product3/2
  Trigonometric Function/1 -> Product3/1
  dY/1 -> Product2/2
  Product2/1 -> Add1/1
  Product3/1 -> Add1/2
  Add1/1 -> dy/1

== system V2x_TC_IntelligentTrafficSigns_cs/BMW_X5_1/Trajectories/ElapsedTrack1/MuxState/Substitute 90 & 270 deg (and its plurals)/Angle 0-360 deg limiter ==
  Angle [deg] : Inport
  Gain : Gain  Gain=1/360
  Gain1 : Gain  Gain=360
  Rounding Function : Rounding
  Sum : Sum  Inputs=|-+
  LimAngle [deg] : Outport
  wires:
  Gain/1 -> Rounding Function/1
  Rounding Function/1 -> Gain1/1
  Gain1/1 -> Sum/1
  Angle [deg]/1 -> Gain/1, Sum/2
  Sum/1 -> LimAngle [deg]/1

== system V2x_TC_IntelligentTrafficSigns_cs/BMW_X5_1/Trajectories/Traj2State1/Traj2State2/Traj2MuxState_Ext/demux ==
  traj : Inport
  Demux : Demux  Outputs=18
  x : Outport
  y : Outport  Port=2
  z : Outport  Port=3
  velx : Outport  Port=4
  vely : Outport  Port=5
  velz : Outport  Port=6
  R : Outport  Port=7
  P : Outport  Port=8
  Y : Outport  Port=9
  velR : Outport  Port=10
  velP : Outport  Port=11
  velY : Outport  Port=12
  accx : Outport  Port=13
  accy : Outport  Port=14
  accz : Outport  Port=15
  accR : Outport  Port=16
  accP : Outport  Port=17
  accY : Outport  Port=18
  wires:
  Demux/18 -> accY/1
  Demux/17 -> accP/1
  Demux/16 -> accR/1
  Demux/15 -> accz/1
  Demux/14 -> accy/1
  Demux/13 -> accx/1
  Demux/12 -> velY/1
  Demux/11 -> velP/1
  Demux/10 -> velR/1
  Demux/9 -> velz/1
  Demux/8 -> vely/1
  Demux/7 -> velx/1
  Demux/6 -> Y/1
  Demux/5 -> P/1
  traj/1 -> Demux/1
  Demux/4 -> R/1
  Demux/3 -> z/1
  Demux/2 -> y/1
  Demux/1 -> x/1

== system V2x_TC_IntelligentTrafficSigns_cs/BMW_X5_1/Traffic Controller/Intersection warning controller/Enabled Subsystem/Intersection/Closest crossing selection/GPS to XYZ difference (vectorized) ==
  hostLat : Inport
  hostLong : Inport  Port=2
  hostAlt : Inport  Port=3
  lat : Inport  Port=4
  long : Inport  Port=5
  alt : Inport  Port=6
  Add : Sum  Inputs=+-
  Add1 : Sum  Inputs=+-
  Add2 : Sum  Inputs=+-
  Add3 : Sum  Inputs=+++
  Product{,1..4} (5 alike) : Product  RndMeth=Zero
  d2r : Gain  Gain=pi/180
  d2r1 : Gain  Gain=pi/180
  mean Earth radius (latitudal) : Constant  Value=6335439.8775
  mean Earth radius (longitudal) : Constant  Value=6378137.55
  dist_sq : Outport
  wires:
  lat/1 -> Add/1
  hostLat/1 -> Add/2
  long/1 -> Add1/1
  hostLong/1 -> Add1/2
  alt/1 -> Add2/1
  hostAlt/1 -> Add2/2
  Add/1 -> d2r/1
  Add1/1 -> d2r1/1
  d2r/1 -> Product2/1
  d2r1/1 -> Product1/1
  mean Earth radius (longitudal)/1 -> Product1/2
  mean Earth radius (latitudal)/1 -> Product2/2
  Product2/1 -> Product/1, Product/2
  Product1/1 -> Product3/1, Product3/2
  Add2/1 -> Product4/2, Product4/1
  Product/1 -> Add3/1
  Product3/1 -> Add3/2
  Product4/1 -> Add3/3
  Add3/1 -> dist_sq/1

== system V2x_TC_IntelligentTrafficSigns_cs/BMW_X5_1/Traffic Controller/Intersection warning controller/Enabled Subsystem/Intersection/Closest crossing selection/If Action Subsystem ==
  min : Inport
  dist_sq : Inport  Port=2
  GPS lat1 : Inport  Port=3
  GPS long1 : Inport  Port=4
  GPS alt1 : Inport  Port=5
  Action Port : ActionPort  PropagateVarSize=During execution  ActionType=then
  Product7 : Product  RndMeth=Zero
  Product8 : Product  RndMeth=Zero
  Product9 : Product  RndMeth=Zero
  Relational Operator3 : RelationalOperator  Operator===  OutDataTypeStr=boolean
  Sum6 : Sum  Inputs=+
  Sum7 : Sum  Inputs=+
  Sum8 : Sum  Inputs=+
  GPS lat : Outport  InitialOutput=0
  GPS long : Outport  Port=2  InitialOutput=0
  GPS alt : Outport  Port=3  InitialOutput=0
  wires:
  min/1 -> Relational Operator3/1
  dist_sq/1 -> Relational Operator3/2
  Relational Operator3/1 -> Product7/1, Product8/1, Product9/1
  GPS lat1/1 -> Product7/2
  GPS long1/1 -> Product8/2
  GPS alt1/1 -> Product9/2
  Product7/1 -> Sum6/1
  Product8/1 -> Sum7/1
  Product9/1 -> Sum8/1
  Sum6/1 -> GPS lat/1
  Sum7/1 -> GPS long/1
  Sum8/1 -> GPS alt/1

== system V2x_TC_IntelligentTrafficSigns_cs/BMW_X5_1/Traffic Controller/Intersection warning controller/Enabled Subsystem/Intersection/Closest crossing selection/If Action Subsystem1 ==
  Action Port : ActionPort  PropagateVarSize=During execution  ActionType=else
  Constant : Constant  Value=0
  Constant1 : Constant  Value=0
  Constant2 : Constant  Value=0
  GPS lat : Outport  InitialOutput=0
  GPS long : Outport  Port=2  InitialOutput=0
  GPS alt : Outport  Port=3  InitialOutput=0
  wires:
  Constant/1 -> GPS lat/1
  Constant1/1 -> GPS long/1
  Constant2/1 -> GPS alt/1

== system V2x_TC_IntelligentTrafficSigns_cs/BMW_X5_1/Traffic Controller/Intersection warning controller/Enabled Subsystem/Intersection/Intersection collision warning controller/For Each Subsystem ==
  actorType : Inport  PortDimensions=1  Partition=on  PartitionDimension=1  PartitionWidth=1
  WM : Inport  Port=2  Partition=off
  Rcross : Inport  Port=3  Partition=off
  target range : Inport  Port=4  Partition=on  PartitionDimension=1  PartitionWidth=1
  target angle : Inport  Port=5  Partition=on  PartitionDimension=1  PartitionWidth=1
  target intention : Inport  Port=6  Partition=on  PartitionDimension=1  PartitionWidth=1
  target heading : Inport  Port=7  Partition=on  PartitionDimension=1  PartitionWidth=1
  targetVelocity : Inport  Port=8  PortDimensions=1  Partition=on  PartitionDimension=1  PartitionWidth=1
  For Each : ForEach  DisableCoverage=on  IndexMode=One-based
  If : If  NumInputs=2  IfExpression=(u1==1 | u1==2 | u1 ==3) & (u2~=0)
  If Action Subsystem : Subsystem  TreatAsAtomicUnit=on
  If Action Subsystem1 : Subsystem  TreatAsAtomicUnit=on
  Merge : Merge
  Merge1 : Merge
  Merge2 : Merge
  warning left lane : Outport  ConcatenationDimension=1
  warning straight lane : Outport  Port=2  ConcatenationDimension=1
  warning right lane : Outport  Port=3  ConcatenationDimension=1
  wires:
  actorType/1 -> If/1
  If/1 -> If Action Subsystem/Action
  If/2 -> If Action Subsystem1/Action
  WM/1 -> If Action Subsystem/1(WM)
  Rcross/1 -> If Action Subsystem/2(Rcross)
  target range/1 -> If Action Subsystem/3(target range)
  target angle/1 -> If Action Subsystem/4(target angle)
  target intention/1 -> If Action Subsystem/5(target intention)
  target heading/1 -> If Action Subsystem/6(target heading)
  If Action Subsystem/1(warning left lane) -> Merge/1
  If Action Subsystem/2(warning straight lane) -> Merge1/1
  If Action Subsystem/3(warning right lane) -> Merge2/1
  If Action Subsystem1/1(warning left lane) -> Merge/2
  If Action Subsystem1/2(warning straight lane) -> Merge1/2
  If Action Subsystem1/3(warning right lane) -> Merge2/2
  Merge/1 -> warning left lane/1
  Merge1/1 -> warning straight lane/1
  Merge2/1 -> warning right lane/1
  targetVelocity/1 -> If/2
